simulink model "ofdm_txrx_supermimo"  (Simulink 7.1)
settings: Solver=VariableStepDiscrete  SolverMode=SingleTasking  StartTime=0.0  StopTime=simOnly_simLength  RelTol=1e-3
PostLoadFcn:
  | ofdm_rx_supermimo_init
InitFcn:
  | ofdm_rx_supermimo_init
StartFcn:
  | ofdm_rx_supermimo_init
values from neighbouring files (symbols this model uses):
  Action0_Config = 0* 31*2^18 + 0*2^24 + PHY_AUTORESPONSE_REQ_GOODHDR + PHY_AUTORESPONSE_REQ_GOODPKT + PHY_AUTORESPONSE_REQ_FLAGA    [ofdm_tx_supermimo_init.m]
  Action1_Config = 0* 62*2^18 + PHY_AUTORESPONSE_REQ_GOODHDR + PHY_AUTORESPONSE_REQ_GOODPKT    [ofdm_tx_supermimo_init.m]
  Action2_Config = 0    [ofdm_tx_supermimo_init.m]
  Action3_Config = 0    [ofdm_tx_supermimo_init.m]
  Action4_Config = 0    [ofdm_tx_supermimo_init.m]
  Action5_Config = 0    [ofdm_tx_supermimo_init.m]
  ActionID_AFTransmit = 31    [ofdm_tx_supermimo_init.m]
  ActionID_Disabled = 0    [ofdm_tx_supermimo_init.m]
  ActionID_SetFlagA = 62    [ofdm_tx_supermimo_init.m]
  ActionID_SetFlagB = 61    [ofdm_tx_supermimo_init.m]
  BER_RAM_init_size = 2048    [ofdm_tx_supermimo_init.m]
  CPLength = 16    [ofdm_tx_supermimo_init.m]
  longCorr_coef_i = [-4*real(LTS) 0]    [ofdm_rx_supermimo_init.m]
  longCorr_coef_q = [4*imag(LTS) 0]    [ofdm_rx_supermimo_init.m]
  Match0_Config = 0    [ofdm_tx_supermimo_init.m]
  Match1_Config = 0    [ofdm_tx_supermimo_init.m]
  Match2_Config = 0    [ofdm_tx_supermimo_init.m]
  Match3_Config = 0    [ofdm_tx_supermimo_init.m]
  Match4_Config = 0    [ofdm_tx_supermimo_init.m]
  Match5_Config = 0    [ofdm_tx_supermimo_init.m]
  Match6_Config = 0    [ofdm_tx_supermimo_init.m]
  Match7_Config = 0    [ofdm_tx_supermimo_init.m]
  max_CP_length = 16    [ofdm_tx_supermimo_init.m]
  max_num_baseRateSymbols = 31    [ofdm_tx_supermimo_init.m]
  max_num_subcarriers = 64    [ofdm_tx_supermimo_init.m]
  max_num_trainingSymbols = 15    [ofdm_tx_supermimo_init.m]
  max_numBytes = 16384    [ofdm_tx_supermimo_init.m]
  max_OFDM_symbols = 511    [ofdm_tx_supermimo_init.m]
  modConstellation_qam16 = .75*[1 3 -1 -3]./3    [ofdm_tx_supermimo_init.m]
  modConstellation_qam64 = 0.875*[3 1 5 7 -3 -1 -5 -7]./7    [ofdm_tx_supermimo_init.m]
  modConstellation_qpsk = [1 -1]./sqrt(2)    [ofdm_tx_supermimo_init.m]
  numBaseRateSymbols = ceil(numHeaderBytes / numBytes_BaseRateOFDMSymbol)    [ofdm_tx_supermimo_init.m]
  numSubcarriers = 64    [ofdm_tx_supermimo_init.m]
  numTrainingSymbols = 2    [ofdm_tx_supermimo_init.m]
  pilot_indicies = 7 + ( (64-21) * 2^8) + ( (64-7) * 2^16) + (21 * 2^24)    (= 356068103)    [ofdm_tx_supermimo_init.m]
  pilotValues = hex2dec('A57D') + (2^16 * hex2dec('5A82'))    [ofdm_tx_supermimo_init.m]
  pkt_crossCorr_thresh = 0.7    [ofdm_rx_supermimo_init.m]
  pkt_energy_thresh = 0    [ofdm_rx_supermimo_init.m]
  pktByteNums = numHeaderBytes +  				byteIndex_numPayloadBytes(1)*2^8 +  				byteIndex_numPayloadBytes(2)*2^16 +  				byteIndex_simpleDynModMasks * 2^24    [ofdm_rx_supermimo_init.m]
  PktDet_Delay = 58+32 +      Symbol_Timing_Offset*2^7 +      0*2^16 +      (117* 2^24)    (= 1962935642)    [ofdm_rx_supermimo_init.m]
  pktTiming_count_payloadStart = pktTiming_count_lsStart + pktTiming_ls_num*pktTiming_ls_length    [ofdm_rx_supermimo_init.m]
  preamble = preamble_scale*[shortsyms_10 longsyms_2]    [ofdm_tx_supermimo_init.m]
  preamble_ROM = preamble_I_ROM + preamble_Q_ROM*2^16    [ofdm_tx_supermimo_init.m]
  RAM_init_size = max_numBytes    (= 16384)    [ofdm_tx_supermimo_init.m]
  reg_AF_Tx_Blanking = (320+80) + ( (320+80+79)*2^16)    (= 31392144)    [ofdm_rx_supermimo_init.m]
  reg_AF_Tx_Scaling = 2944    [ofdm_rx_supermimo_init.m]
  reg_Interrupt_PktBuf_Control = reg_InterruptControl +  	1 * 2^16 +  	0 * 2^24    (= 65656)    [ofdm_rx_supermimo_init.m]
  reg_PilotCalcParams = (4 * 2^16) + (minPilotChanMag)    (= 262184.96)    [ofdm_rx_supermimo_init.m]
  regRx_chanEst_minMag = ((0.5)*2^15) + ((0.5 * 2^15)*2^16)    (= 1073758208)    [ofdm_rx_supermimo_init.m]
  regRx_coarseCalcCorrection = round((1.3e-3 - 1.2982e-3)*2^32)    [ofdm_rx_supermimo_init.m]
  regRx_pilotCalcCorrection = round(1.00115 * 2^31)    [ofdm_rx_supermimo_init.m]
  regRx_preCFOoptions = 0 * 2^0 +  	1 * 2^1 +  	0    (= 2)    [ofdm_rx_supermimo_init.m]
  rx_controlBits = 1 * 2  		 + 1 * 4  		 + 0 * 8  		 + 1 * 16 * rx_SISO_Mode  		 + 1 * 32  		 + 1 * 64  		 + 1 * 128  		 + 1 * 256  		 + 1 * 512  		 + 1 * 1024  		 + 1 * 4096  		 + 0 * 16384  		 + 0 * 32768  		 + 1 * 65536  		 + 0 * 131072  		 + 0 * 262144  		 + 1 * 524288  		 + 1 * 1048576 * tx_Alamouti_Mode  		 + 0 * 2097152           + 1 * 33554432  		 + 0 * 4194304  		 + 0 * 8388608  		 + 0 * 16777216  		 + 1 *  ...(+49 chars)    [ofdm_rx_supermimo_init.m]
  Rx_PktDet_LongCorr_Params = LongCorrCounterLoad + 0*2^8 + 0*2^16 + 255*2^24    (= 4278190325)    [ofdm_rx_supermimo_init.m]
  Rx_PktDet_LongCorrThresholds = 8000    [ofdm_rx_supermimo_init.m]
  rx_postEq_scaling = round(rxScaling*2^11) * (1 + 2^16)    [ofdm_rx_supermimo_init.m]
  RxDataScrambling_Seq = [40 198 78 63 82 173 102 245 48 111 172 115 147 230 216 93 72 65 62 2 205 242 122 90 128 83 105 97 73 10 5 252]    [ofdm_tx_supermimo_init.m]
  RxReg_FixedPktLen = 0    [ofdm_rx_supermimo_init.m]
  shortSymbol_time = shortSymbol_time(1:16).'    [ofdm_tx_supermimo_init.m]
  simOnly_numSamples = length(preamble)+( (numSubcarriers+CPLength)*(numTrainingSymbols + numBaseRateSymbols + numFullRateSymbols/2) )    [ofdm_tx_supermimo_init.m]
  train = [train train]    [ofdm_tx_supermimo_init.m]
  tx_controlBits = (antB_preambleShift * 2^4) + (2^1 * tx_Alamouti_Mode) +  	(2^0 * tx_SISO_Mode) + 4*0 + 8*1 + 0*256 + 512*0 + 1024*0 +  	0*hex2dec('1000') + 0*hex2dec('2000') + 0*hex2dec('4000') +  	1*hex2dec('8000') + 1*hex2dec('10000') + hex2dec('20000') +  	16*hex2dec('100000')    [ofdm_tx_supermimo_init.m]
  tx_delays = (50*2^0) + (0*2^8) + (30*2^12)    (= 122930)    [ofdm_tx_supermimo_init.m]
  tx_scaling = (3072) + (12288 * 2^16)    (= 805309440)    [ofdm_tx_supermimo_init.m]
  TxDataScrambling_Seq = [40 198 78 63 82 173 102 245 48 111 172 115 147 230 216 93 72 65 62 2 205 242 122 90 128 83 105 97 73 10 5 252]    [ofdm_tx_supermimo_init.m]
  txfilt_firpm = 0.95*txfilt_firpm./(max(txfilt_firpm))    [ofdm_tx_supermimo_init.m]
  txfilt_intfilt = [0 intfilt(4,4,.8)]    [ofdm_tx_supermimo_init.m]
  txReg_symbolCounts = (2^8 *numBaseRateSymbols ) + numTrainingSymbols    [ofdm_tx_supermimo_init.m]
  TxRx_FFTScaling = (3 * 2^0) +  	(2 * 2^2) +  	(1 * 2^4) +  	(1 * 2^6) +  	(1 * 2^8) +  	(0 * 2^10)    (= 347)    [ofdm_tx_supermimo_init.m]
undefined symbols (not defined in the file or in its folder): AntA, AntB, BaseRate, Down, gcbh, numBuffs, numBytes, Up, xlBlockLoadCallback, xlGetSimulinkPeriod, xlipgetpartsetting
libraries: xbsIndex_r4 x6035, simulink x4, dspsigops x4, commchan3 x2, dspsrcs4 x2, commrflib2 x2
(part 40 of 61)

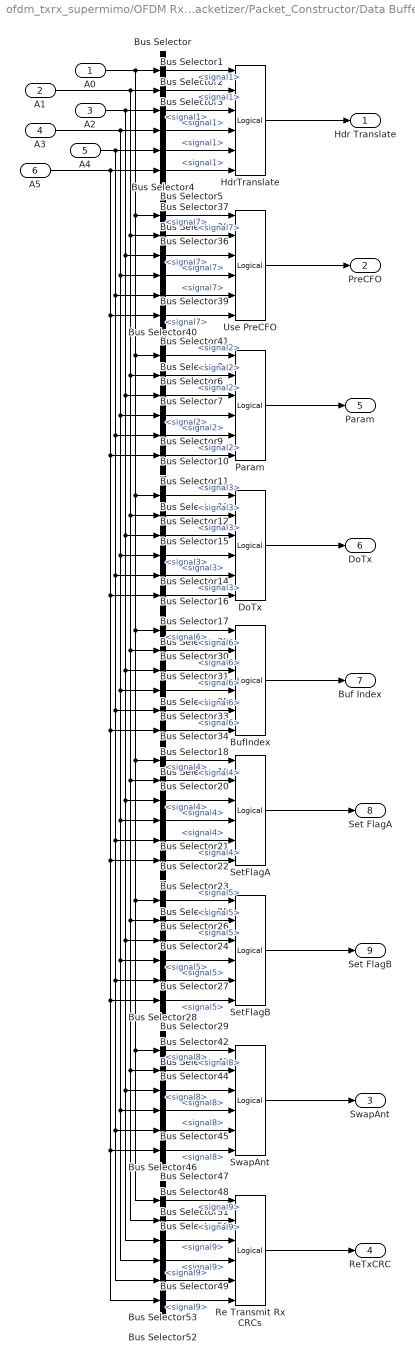
== system ofdm_txrx_supermimo/OFDM Rx MIMO/Equalizer & Packetizer/Packet_Constructor/Data Buffer/AutoResponse Processing/Action Combining ==
  A0 : Inport
  A1 : Inport  Port=2
  A2 : Inport  Port=3
  A3 : Inport  Port=4
  A4 : Inport  Port=5
  A5 : Inport  Port=6
  BufIndex : xbsIndex_r4/Logical  logical_function=OR  inputs=6  en=off  latency=0  precision=Full  arith_type=Unsigned  n_bits=16  bin_pt=0  align_bp=on  dbl_ovrd=off  block_type=logical
  Bus Selector{,1..5} (6 alike) : BusSelector  OutputSignals=signal1
  Bus Selector{6..11} (6 alike) : BusSelector  OutputSignals=signal2
  Bus Selector{12..17} (6 alike) : BusSelector  OutputSignals=signal3
  Bus Selector{18..23} (6 alike) : BusSelector  OutputSignals=signal4
  Bus Selector{24..29} (6 alike) : BusSelector  OutputSignals=signal5
  Bus Selector{30..35} (6 alike) : BusSelector  OutputSignals=signal6
  Bus Selector{36..41} (6 alike) : BusSelector  OutputSignals=signal7
  Bus Selector{42..47} (6 alike) : BusSelector  OutputSignals=signal8
  Bus Selector{48..53} (6 alike) : BusSelector  OutputSignals=signal9
  "DoTx " : xbsIndex_r4/Logical  logical_function=OR  inputs=6  en=off  latency=0  precision=Full  arith_type=Unsigned  n_bits=16  bin_pt=0  align_bp=on  dbl_ovrd=off  block_type=logical
  HdrTranslate : xbsIndex_r4/Logical  logical_function=OR  inputs=6  en=off  latency=0  precision=Full  arith_type=Unsigned  n_bits=16  bin_pt=0  align_bp=on  dbl_ovrd=off  block_type=logical
  "Param " : xbsIndex_r4/Logical  logical_function=OR  inputs=6  en=off  latency=0  precision=Full  arith_type=Unsigned  n_bits=16  bin_pt=0  align_bp=on  dbl_ovrd=off  block_type=logical
  Re Transmit Rx CRCs : xbsIndex_r4/Logical  logical_function=OR  inputs=6  en=off  latency=0  precision=Full  arith_type=Unsigned  n_bits=16  bin_pt=0  align_bp=on  dbl_ovrd=off  block_type=logical
  "SetFlagA " : xbsIndex_r4/Logical  logical_function=OR  inputs=6  en=off  latency=0  precision=Full  arith_type=Unsigned  n_bits=16  bin_pt=0  align_bp=on  dbl_ovrd=off  block_type=logical
  SetFlagB : xbsIndex_r4/Logical  logical_function=OR  inputs=6  en=off  latency=0  precision=Full  arith_type=Unsigned  n_bits=16  bin_pt=0  align_bp=on  dbl_ovrd=off  block_type=logical
  SwapAnt : xbsIndex_r4/Logical  logical_function=OR  inputs=6  en=off  latency=0  precision=Full  arith_type=Unsigned  n_bits=16  bin_pt=0  align_bp=on  dbl_ovrd=off  block_type=logical
  Use PreCFO : xbsIndex_r4/Logical  logical_function=OR  inputs=6  en=off  latency=0  precision=Full  arith_type=Unsigned  n_bits=16  bin_pt=0  align_bp=on  dbl_ovrd=off  block_type=logical
  Hdr Translate : Outport
  PreCFO : Outport  Port=2
  " SwapAnt" : Outport  Port=3
  ReTxCRC : Outport  Port=4
  Param : Outport  Port=5
  DoTx : Outport  Port=6
  Buf Index : Outport  Port=7
  Set FlagA : Outport  Port=8
  Set FlagB : Outport  Port=9
  wires:
  A3/1 -> Bus Selector3/1, Bus Selector9/1, Bus Selector33/1, Bus Selector21/1, Bus Selector27/1, Bus Selector45/1, Bus Selector49/1, Bus Selector14/1, Bus Selector39/1
  A4/1 -> Bus Selector4/1, Bus Selector10/1, Bus Selector34/1, Bus Selector22/1, Bus Selector28/1, Bus Selector46/1, Bus Selector53/1, Bus Selector16/1, Bus Selector40/1
  A5/1 -> Bus Selector5/1, Bus Selector41/1, Bus Selector11/1, Bus Selector17/1, Bus Selector35/1, Bus Selector23/1, Bus Selector29/1, Bus Selector47/1, Bus Selector52/1
  Bus Selector29/1 -> SetFlagB/6  "<signal5>"
  Bus Selector39/1 -> Use PreCFO/4  "<signal7>"
  Bus Selector40/1 -> Use PreCFO/5  "<signal7>"
  Bus Selector41/1 -> Use PreCFO/6  "<signal7>"
  Bus Selector38/1 -> Use PreCFO/3  "<signal7>"
  Bus Selector37/1 -> Use PreCFO/2  "<signal7>"
  Bus Selector36/1 -> Use PreCFO/1  "<signal7>"
  Bus Selector35/1 -> BufIndex/6  "<signal6>"
  Bus Selector34/1 -> BufIndex/5  "<signal6>"
  Bus Selector33/1 -> BufIndex/4  "<signal6>"
  Bus Selector32/1 -> BufIndex/3  "<signal6>"
  Bus Selector31/1 -> BufIndex/2  "<signal6>"
  Bus Selector30/1 -> BufIndex/1  "<signal6>"
  Bus Selector28/1 -> SetFlagB/5  "<signal5>"
  Bus Selector27/1 -> SetFlagB/4  "<signal5>"
  Bus Selector25/1 -> SetFlagB/3  "<signal5>"
  Bus Selector24/1 -> SetFlagB/2  "<signal5>"
  Bus Selector26/1 -> SetFlagB/1  "<signal5>"
  Bus Selector23/1 -> "SetFlagA "/6  "<signal4>"
  Bus Selector22/1 -> "SetFlagA "/5  "<signal4>"
  Bus Selector21/1 -> "SetFlagA "/4  "<signal4>"
  Bus Selector19/1 -> "SetFlagA "/3  "<signal4>"
  Bus Selector18/1 -> "SetFlagA "/2  "<signal4>"
  Bus Selector20/1 -> "SetFlagA "/1  "<signal4>"
  Bus Selector17/1 -> "DoTx "/6  "<signal3>"
  Bus Selector16/1 -> "DoTx "/5  "<signal3>"
  Bus Selector14/1 -> "DoTx "/4  "<signal3>"
  Bus Selector13/1 -> "DoTx "/3  "<signal3>"
  Bus Selector15/1 -> "DoTx "/2  "<signal3>"
  Bus Selector12/1 -> "DoTx "/1  "<signal3>"
  Bus Selector11/1 -> "Param "/6  "<signal2>"
  Bus Selector10/1 -> "Param "/5  "<signal2>"
  Bus Selector9/1 -> "Param "/4  "<signal2>"
  Bus Selector8/1 -> "Param "/3  "<signal2>"
  Bus Selector7/1 -> "Param "/2  "<signal2>"
  Bus Selector6/1 -> "Param "/1  "<signal2>"
  Bus Selector5/1 -> HdrTranslate/6  "<signal1>"
  Bus Selector4/1 -> HdrTranslate/5  "<signal1>"
  Bus Selector3/1 -> HdrTranslate/4  "<signal1>"
  Bus Selector2/1 -> HdrTranslate/3  "<signal1>"
  Bus Selector1/1 -> HdrTranslate/2  "<signal1>"
  Bus Selector/1 -> HdrTranslate/1  "<signal1>"
  A2/1 -> Bus Selector38/1, Bus Selector8/1, Bus Selector32/1, Bus Selector19/1, Bus Selector25/1, Bus Selector43/1, Bus Selector51/1, Bus Selector13/1, Bus Selector2/1
  A1/1 -> Bus Selector37/1, Bus Selector7/1, Bus Selector31/1, Bus Selector18/1, Bus Selector24/1, Bus Selector42/1, Bus Selector48/1, Bus Selector15/1, Bus Selector1/1
  A0/1 -> Bus Selector36/1, Bus Selector30/1, Bus Selector20/1, Bus Selector26/1, Bus Selector44/1, Bus Selector50/1, Bus Selector12/1, Bus Selector6/1, Bus Selector/1
  HdrTranslate/1 -> Hdr Translate/1
  Use PreCFO/1 -> PreCFO/1
  "Param "/1 -> Param/1
  "DoTx "/1 -> DoTx/1
  BufIndex/1 -> Buf Index/1
  "SetFlagA "/1 -> Set FlagA/1
  SetFlagB/1 -> Set FlagB/1
  Bus Selector47/1 -> SwapAnt/6  "<signal8>"
  Bus Selector46/1 -> SwapAnt/5  "<signal8>"
  Bus Selector45/1 -> SwapAnt/4  "<signal8>"
  Bus Selector43/1 -> SwapAnt/3  "<signal8>"
  Bus Selector42/1 -> SwapAnt/2  "<signal8>"
  Bus Selector44/1 -> SwapAnt/1  "<signal8>"
  SwapAnt/1 -> " SwapAnt"/1
  Bus Selector50/1 -> Re Transmit Rx CRCs/1  "<signal9>"
  Re Transmit Rx CRCs/1 -> ReTxCRC/1
  Bus Selector48/1 -> Re Transmit Rx CRCs/2  "<signal9>"
  Bus Selector51/1 -> Re Transmit Rx CRCs/3  "<signal9>"
  Bus Selector49/1 -> Re Transmit Rx CRCs/4  "<signal9>"
  Bus Selector53/1 -> Re Transmit Rx CRCs/5  "<signal9>"
  Bus Selector52/1 -> Re Transmit Rx CRCs/6  "<signal9>"

== system ofdm_txrx_supermimo/OFDM Rx MIMO/Equalizer & Packetizer/Packet_Constructor/Data Buffer/AutoResponse Processing/Action Decoding ==
  Match : Inport
  FlagA : Inport  Port=2
  FlagB : Inport  Port=3
  Action0 : Subsystem
  Action1 : Subsystem
  Action2 : Subsystem
  Action3 : Subsystem
  Action4 : Subsystem
  Action5 : Subsystem
  Bus Creator{,1..5} (6 alike) : BusCreator  Inputs=9
  Concat : xbsIndex_r4/Concat  num_inputs=3  dbl_ovrd=off  block_type=concat
      settings: block_version=10.1.3
  " Action0" : Outport
  " Action1" : Outport  Port=2
  " Action2" : Outport  Port=3
  " Action3" : Outport  Port=4
  " Action4" : Outport  Port=5
  " Action5" : Outport  Port=6
  tag scope: RxAutoReply_MatchStatus=global
  wires:
  Match/1 -> Action0/1(Match), Action4/1(Match), Action5/1(Match), Action3/1(Match), Action2/1(Match), Action1/1(Match), Concat/3
  Action0/1(UseTrans) -> Bus Creator/1
  Action0/2(Param) -> Bus Creator/2
  Action0/3(DoTx) -> Bus Creator/3
  Action0/4(Set_FlagA) -> Bus Creator/4
  Action0/5(Set_FlagB) -> Bus Creator/5
  Bus Creator/1 -> " Action0"/1
  FlagA/1 -> Action0/2(FlagA), Action4/2(FlagA), Action5/2(FlagA), Action3/2(FlagA), Action2/2(FlagA), Action1/2(FlagA), Concat/1
  FlagB/1 -> Action4/3(FlagB), Action5/3(FlagB), Action3/3(FlagB), Action2/3(FlagB), Action1/3(FlagB), Action0/3(FlagB), Concat/2
  Action0/6(TxBufNum) -> Bus Creator/6
  Action0/7(UsePreCFO) -> Bus Creator/7
  Concat/1 -> [RxAutoReply_MatchStatus]
  Action0/8(SwapAnt) -> Bus Creator/8
  Action0/9(ReTxCRC) -> Bus Creator/9
  Action1/1(UseTrans) -> Bus Creator1/1
  Action1/2(Param) -> Bus Creator1/2
  Action1/3(DoTx) -> Bus Creator1/3
  Action1/4(Set_FlagA) -> Bus Creator1/4
  Action1/5(Set_FlagB) -> Bus Creator1/5
  Bus Creator1/1 -> " Action1"/1
  Action1/6(TxBufNum) -> Bus Creator1/6
  Action1/7(UsePreCFO) -> Bus Creator1/7
  Action1/8(SwapAnt) -> Bus Creator1/8
  Action1/9(ReTxCRC) -> Bus Creator1/9
  Action2/1(UseTrans) -> Bus Creator2/1
  Action2/2(Param) -> Bus Creator2/2
  Action2/3(DoTx) -> Bus Creator2/3
  Action2/4(Set_FlagA) -> Bus Creator2/4
  Action2/5(Set_FlagB) -> Bus Creator2/5
  Bus Creator2/1 -> " Action2"/1
  Action2/6(TxBufNum) -> Bus Creator2/6
  Action2/7(UsePreCFO) -> Bus Creator2/7
  Action2/8(SwapAnt) -> Bus Creator2/8
  Action2/9(ReTxCRC) -> Bus Creator2/9
  Action3/1(UseTrans) -> Bus Creator3/1
  Action3/2(Param) -> Bus Creator3/2
  Action3/3(DoTx) -> Bus Creator3/3
  Action3/4(Set_FlagA) -> Bus Creator3/4
  Action3/5(Set_FlagB) -> Bus Creator3/5
  Bus Creator3/1 -> " Action3"/1
  Action3/6(TxBufNum) -> Bus Creator3/6
  Action3/7(UsePreCFO) -> Bus Creator3/7
  Action3/8(SwapAnt) -> Bus Creator3/8
  Action3/9(ReTxCRC) -> Bus Creator3/9
  Action4/1(UseTrans) -> Bus Creator4/1
  Action4/2(Param) -> Bus Creator4/2
  Action4/3(DoTx) -> Bus Creator4/3
  Action4/4(Set_FlagA) -> Bus Creator4/4
  Action4/5(Set_FlagB) -> Bus Creator4/5
  Bus Creator4/1 -> " Action4"/1
  Action4/6(TxBufNum) -> Bus Creator4/6
  Action4/7(UsePreCFO) -> Bus Creator4/7
  Action4/8(SwapAnt) -> Bus Creator4/8
  Action4/9(ReTxCRC) -> Bus Creator4/9
  Action5/1(UseTrans) -> Bus Creator5/1
  Action5/2(Param) -> Bus Creator5/2
  Action5/3(DoTx) -> Bus Creator5/3
  Action5/4(Set_FlagA) -> Bus Creator5/4
  Action5/5(Set_FlagB) -> Bus Creator5/5
  Bus Creator5/1 -> " Action5"/1
  Action5/6(TxBufNum) -> Bus Creator5/6
  Action5/7(UsePreCFO) -> Bus Creator5/7
  Action5/8(SwapAnt) -> Bus Creator5/8
  Action5/9(ReTxCRC) -> Bus Creator5/9

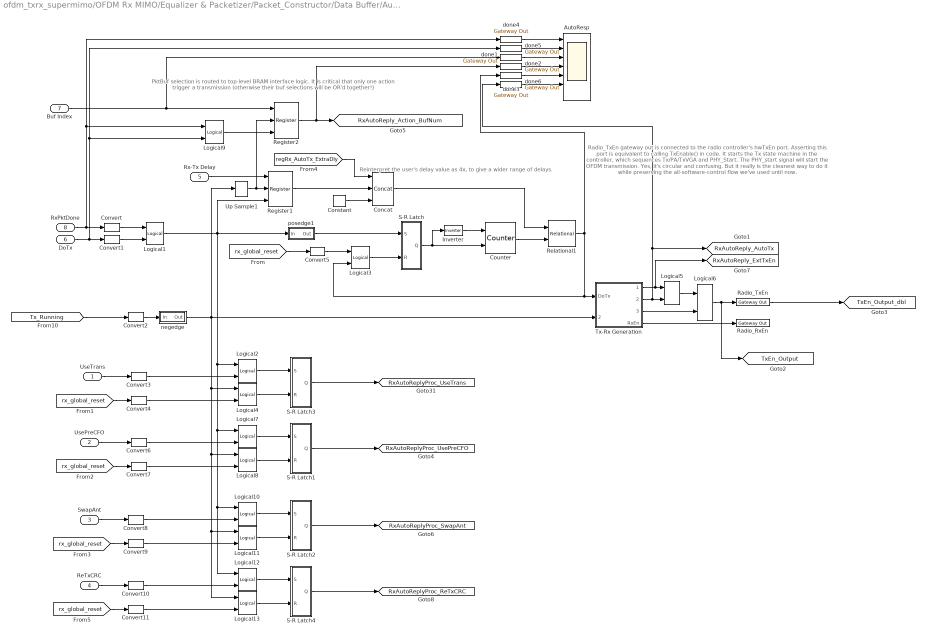
== system ofdm_txrx_supermimo/OFDM Rx MIMO/Equalizer & Packetizer/Packet_Constructor/Data Buffer/AutoResponse Processing/AutoResponse I/O ==
  note: Radio_TxEn gateway out is connected to the radio controller's hwTxEn port.
      | Asserting this port is equivalent to calling TxEnable() in code. It starts the Tx
      | state machine in the controller, which sequences Tx/PA/TxVGA and PHY_Start.
      | The PHY_start signal will start the OFDM transmission.
      |
      | Yes, it's circular and confusing. But it really is the cleanest way to do it while
      | preserving the all-software-control flow we've used until now.
  note: PktBuf selection is routed to top-level
      | BRAM interface logic. It is critical that only
      | one action trigger a transmission (otherwise
      | their buf selections will be OR'd together!)
  note: Reinterpret the user's delay value as 4x,
      | to give a wider range of delays.
  UseTrans : Inport  OutDataType=sfix(16)  OutScaling=2^0
  UsePreCFO : Inport  Port=2  OutDataType=sfix(16)  OutScaling=2^0
  SwapAnt : Inport  Port=3  OutDataType=sfix(16)  OutScaling=2^0
  ReTxCRC : Inport  Port=4  OutDataType=sfix(16)  OutScaling=2^0
  Rx-Tx Delay : Inport  Port=5  OutDataType=sfix(16)  OutScaling=2^0
  DoTx : Inport  Port=6  OutDataType=sfix(16)  OutScaling=2^0
  Buf Index : Inport  Port=7  OutDataType=sfix(16)  OutScaling=2^0
  RxPktDone : Inport  Port=8  OutDataType=sfix(16)  OutScaling=2^0
  AutoResp : Scope  NumInputPorts=7
      settings: TimeRange=392.7801724137931  YMin=-0.5~-0.25~-1~-1~0~0~0  YMax=0.5~0.15~1~1~1~3500~1  SaveName=ScopeData25
  Concat : xbsIndex_r4/Concat  num_inputs=3  dbl_ovrd=off  block_type=concat
      settings: block_version=10.1.3
  Constant : xbsIndex_r4/Constant  arith_type=Unsigned  const=0  n_bits=1  bin_pt=0  explicit_period=off  period=1  equ=P=C  opselect=C  inp2=PCIN>>17  opr=+  inp1=P  carry=CIN  dbl_ovrd=off  block_type=constant
  Convert{,1..11} (12 alike) : xbsIndex_r4/Convert  arith_type=Boolean  n_bits=16  bin_pt=14  quantization=Truncate  overflow=Wrap  latency=0  dbl_ovrd=off  pipeline=off  block_type=convert
      settings: block_version=10.1.3
  Counter : xbsIndex_r4/Counter  cnt_type=Free Running  cnt_to=0  operation=Up  start_count=0  cnt_by_val=1  arith_type=Unsigned  n_bits=14  bin_pt=0  load_pin=off  rst=on  en=on  explicit_period=off  period=4  dbl_ovrd=off  use_behavioral_HDL=off  use_rpm=off  implementation=Fabric  block_type=counter
      settings: block_version=VER_STRING_GOES_HERE
  Inverter : xbsIndex_r4/Inverter  en=off  latency=0  dbl_ovrd=off  block_type=inv
      settings: xl_area=[0 0 0 0 0 0 0]  block_version=8.2
  Logical{1..2,7,9..10,12} (6 alike) : xbsIndex_r4/Logical  logical_function=AND  inputs=2  en=off  latency=0  precision=Full  arith_type=Unsigned  n_bits=16  bin_pt=0  align_bp=on  dbl_ovrd=off  block_type=logical
  Logical{3..6,8,11,13} (7 alike) : xbsIndex_r4/Logical  logical_function=OR  inputs=2  en=off  latency=0  precision=Full  arith_type=Unsigned  n_bits=16  bin_pt=0  align_bp=on  dbl_ovrd=off  block_type=logical
  Radio_RxEn : xbsIndex_r4/Gateway Out  hdl_port=on  timing_constraint=None  locs_specified=off  LOCs={}  block_type=gatewayout
      settings: infoedit="Gateway out block.  Converts Xilinx fixed point inputs into ouputs of type Simulink integer, double, or fixed point.<P><P>Hardware notes:  In hardware these blocks become top level output ports or are discarded, depending on how they are configured."  block_version=10.1.3
  Radio_TxEn : xbsIndex_r4/Gateway Out  hdl_port=on  timing_constraint=None  locs_specified=off  LOCs={}  block_type=gatewayout
      settings: infoedit="Gateway out block.  Converts Xilinx fixed point inputs into ouputs of type Simulink integer, double, or fixed point.<P><P>Hardware notes:  In hardware these blocks become top level output ports or are discarded, depending on how they are configured."  block_version=10.1.3
  Register1 : xbsIndex_r4/Register  init=0  rst=on  en=on  dbl_ovrd=off  block_type=register
      settings: xl_area=[5 10 0 0 0 0 0]  block_version=VER_STRING_GOES_HERE
  Register2 : xbsIndex_r4/Register  init=0  rst=on  en=on  dbl_ovrd=off  block_type=register
      settings: xl_area=[5 10 0 0 0 0 0]  block_version=VER_STRING_GOES_HERE
  Relational1 : xbsIndex_r4/Relational  mode=a<b  en=off  latency=0  dbl_ovrd=off  block_type=relational
      settings: block_version=VER_STRING_GOES_HERE
  S-R Latch : Subsystem
  S-R Latch1 : Subsystem
  S-R Latch2 : Subsystem
  S-R Latch3 : Subsystem
  S-R Latch4 : Subsystem
  Tx-Rx Generation : Subsystem
  Up Sample1 : xbsIndex_r4/Up Sample  sample_ratio=2  copy_samples=on  dbl_ovrd=off  block_type=usamp
      settings: infoedit="Up samples input data.  Inserted values  can be zeros or copies of the most recent input sample.<P><P>Hardware notes: No hardware is needed if inserted values are copies of the input sample; otherwise, a mux and single bit flip-flop are used."  block_version=10.1.3
  done{1..6} (6 alike) : xbsIndex_r4/Gateway Out  hdl_port=off  timing_constraint=None  locs_specified=off  LOCs={}  block_type=gatewayout
      settings: infoedit="Gateway out block.  Converts Xilinx fixed point inputs into ouputs of type Simulink integer, double, or fixed point.<P><P>Hardware notes:  In hardware these blocks become top level output ports or are discarded, depending on how they are configured."  xl_area=[0 0 0 0 1 0 0]  block_version=VER_STRING_GOES_HERE
  negedge : Subsystem
  posedge1 : Subsystem
  tag scope: RxAutoReplyProc_ReTxCRC=global, RxAutoReplyProc_SwapAnt=global, RxAutoReplyProc_UsePreCFO=global, RxAutoReplyProc_UseTrans=global, RxAutoReply_Action_BufNum=global, RxAutoReply_AutoTx=global, RxAutoReply_ExtTxEn=global, TxEn_Output=global, TxEn_Output_dbl=global
  wires:
  S-R Latch/1(Q) -> Counter/2, Inverter/1
  negedge/1(Out) -> Up Sample1/1, Logical4/1, Logical8/1, Logical11/1, Logical13/1, Tx-Rx Generation/2(TxDone)
  [Tx_Running] -> Convert2/1
  posedge1/1(Out) -> S-R Latch/1(S)
  Inverter/1 -> Counter/1
  Tx-Rx Generation/4(RxEn) -> Radio_RxEn/1
  Relational1/1 -> Logical3/2, Tx-Rx Generation/1(DoTx), done3/1
  Logical3/1 -> S-R Latch/2(R)
  [rx_global_reset] -> Convert5/1
  Logical1/1 -> posedge1/1(In), Register1/3, Logical2/1, Logical7/1, Logical10/1, Logical12/1
  Logical2/1 -> S-R Latch3/1(S)
  S-R Latch3/1(Q) -> [RxAutoReplyProc_UseTrans]
  Radio_TxEn/1 -> [TxEn_Output_dbl]
  DoTx/1 -> Convert1/1, Logical9/2, done5/1
  RxPktDone/1 -> Convert/1, Logical9/1, done4/1
  UseTrans/1 -> Convert3/1
  [rx_global_reset] -> Convert4/1
  Logical4/1 -> S-R Latch3/2(R)
  Convert/1 -> Logical1/1
  Convert1/1 -> Logical1/2
  Convert2/1 -> negedge/1(In)
  Convert3/1 -> Logical2/2
  Convert4/1 -> Logical4/2
  Convert5/1 -> Logical3/1
  Tx-Rx Generation/2(AutoTxEn) -> Logical5/2, [RxAutoReply_AutoTx], done6/1
  Logical6/1 -> Radio_TxEn/1, [TxEn_Output]
  Tx-Rx Generation/3(UserTxEn) -> Logical6/2
  Tx-Rx Generation/1(ExtTxEn) -> Logical5/1, [RxAutoReply_ExtTxEn]
  Logical5/1 -> Logical6/1
  Rx-Tx Delay/1 -> Register1/1
  Up Sample1/1 -> Register1/2, Register2/2
  Logical7/1 -> S-R Latch1/1(S)
  S-R Latch1/1(Q) -> [RxAutoReplyProc_UsePreCFO]
  UsePreCFO/1 -> Convert6/1
  [rx_global_reset] -> Convert7/1
  Logical8/1 -> S-R Latch1/2(R)
  Convert6/1 -> Logical7/2
  Convert7/1 -> Logical8/2
  Logical9/1 -> Register2/3
  Buf Index/1 -> Register2/1, done1/1
  Register2/1 -> [RxAutoReply_Action_BufNum], done2/1
  Constant/1 -> Concat/3
  Register1/1 -> Concat/2
  Concat/1 -> Relational1/1
  Counter/1 -> Relational1/2
  SwapAnt/1 -> Convert8/1
  Logical10/1 -> S-R Latch2/1(S)
  Logical11/1 -> S-R Latch2/2(R)
  Convert8/1 -> Logical10/2
  [rx_global_reset] -> Convert9/1
  Convert9/1 -> Logical11/2
  S-R Latch2/1(Q) -> [RxAutoReplyProc_SwapAnt]
  [regRx_AutoTx_ExtraDly] -> Concat/1
  done4/1 -> AutoResp/1
  done5/1 -> AutoResp/2
  done1/1 -> AutoResp/3
  done2/1 -> AutoResp/4
  done3/1 -> AutoResp/5
  done6/1 -> AutoResp/6
  ReTxCRC/1 -> Convert10/1
  Logical12/1 -> S-R Latch4/1(S)
  Logical13/1 -> S-R Latch4/2(R)
  Convert10/1 -> Logical12/2
  [rx_global_reset] -> Convert11/1
  Convert11/1 -> Logical13/2
  S-R Latch4/1(Q) -> [RxAutoReplyProc_ReTxCRC]

== system ofdm_txrx_supermimo/OFDM Rx MIMO/Equalizer & Packetizer/Packet_Constructor/Data Buffer/AutoResponse Processing/Flag A ==
  note: The flag is cleared after every packet reception. The clear is
      | delayed 2 cycles so the matching logic can use the flag set by the
      | previous packet in combination with header/status values
      | from the packet that just finished.
      |
      | The flag is set 1 cycle later if some action asserts the SetFlag input.
  SetFlag : Inport
  PktDone : Inport  Port=2
  Delay : xbsIndex_r4/Delay  en=off  latency=2  dbl_ovrd=off  reg_retiming=off  block_type=delay
  Delay1 : xbsIndex_r4/Delay  en=off  latency=1  dbl_ovrd=off  reg_retiming=off  block_type=delay
  Flag A Latch : Subsystem
  Logical1 : xbsIndex_r4/Logical  logical_function=AND  inputs=2  en=off  latency=0  precision=Full  arith_type=Unsigned  n_bits=16  bin_pt=0  align_bp=on  dbl_ovrd=off  block_type=logical
  Logical2 : xbsIndex_r4/Logical  logical_function=OR  inputs=3  en=off  latency=0  precision=Full  arith_type=Unsigned  n_bits=16  bin_pt=0  align_bp=on  dbl_ovrd=off  block_type=logical
  Flag : Outport
  wires:
  [rx_global_reset] -> Logical2/2
  Logical2/1 -> Flag A Latch/2(R)
  Delay/1 -> Logical2/1, Delay1/1
  Logical1/1 -> Flag A Latch/1(S)
  Flag A Latch/1(Q) -> Flag/1
  PktDone/1 -> Delay/1
  SetFlag/1 -> Logical1/1
  Delay1/1 -> Logical1/2
  [RxReg_ResetFlagA] -> Logical2/3

== system ofdm_txrx_supermimo/OFDM Rx MIMO/Equalizer & Packetizer/Packet_Constructor/Data Buffer/AutoResponse Processing/Flag B ==
  note: The flag is cleared after every packet reception. The clear is
      | delayed 2 cycles so the matching logic can use the flag set by the
      | previous packet in combination with header/status values
      | from the packet that just finished.
      |
      | The flag is set 1 cycle later if some action asserts the SetFlag input.
  SetFlag : Inport
  PktDone : Inport  Port=2
  Delay : xbsIndex_r4/Delay  en=off  latency=2  dbl_ovrd=off  reg_retiming=off  block_type=delay
  Delay1 : xbsIndex_r4/Delay  en=off  latency=1  dbl_ovrd=off  reg_retiming=off  block_type=delay
  Flag B Latch : Subsystem
  Logical1 : xbsIndex_r4/Logical  logical_function=AND  inputs=2  en=off  latency=0  precision=Full  arith_type=Unsigned  n_bits=16  bin_pt=0  align_bp=on  dbl_ovrd=off  block_type=logical
  Logical2 : xbsIndex_r4/Logical  logical_function=OR  inputs=3  en=off  latency=0  precision=Full  arith_type=Unsigned  n_bits=16  bin_pt=0  align_bp=on  dbl_ovrd=off  block_type=logical
  Flag : Outport
  wires:
  [RxReg_ResetFlagB] -> Logical2/3
  Delay1/1 -> Logical1/2
  SetFlag/1 -> Logical1/1
  PktDone/1 -> Delay/1
  Flag B Latch/1(Q) -> Flag/1
  Logical1/1 -> Flag B Latch/1(S)
  Delay/1 -> Delay1/1, Logical2/1
  Logical2/1 -> Flag B Latch/2(R)
  [rx_global_reset] -> Logical2/2

== system ofdm_txrx_supermimo/OFDM Rx MIMO/Equalizer & Packetizer/Packet_Constructor/Data Buffer/AutoResponse Processing/Header Byte Matching ==
  RxByteAddr : Inport  OutDataType=sfix(16)  OutScaling=2^0
  RxByte : Inport  Port=2  OutDataType=sfix(16)  OutScaling=2^0
  RxByteWE : Inport  Port=3  OutDataType=sfix(16)  OutScaling=2^0
  Rst : Inport  Port=4  OutDataType=sfix(16)  OutScaling=2^0
  Concat : xbsIndex_r4/Concat  num_inputs=8  dbl_ovrd=off  block_type=concat
      settings: block_version=10.1.3
  Constant : xbsIndex_r4/Constant  arith_type=Unsigned  const=0  n_bits=1  bin_pt=0  explicit_period=off  period=1  equ=P=C  opselect=C  inp2=PCIN>>17  opr=+  inp1=P  carry=CIN  dbl_ovrd=off  block_type=constant
  Field Slices : Subsystem
  Field Slices1 : Subsystem
  Field Slices2 : Subsystem
  Field Slices3 : Subsystem
  Field Slices4 : Subsystem
  Field Slices5 : Subsystem
  Match0 : Subsystem
  Match1 : Subsystem
  Match2 : Subsystem
  Match3 : Subsystem
  Match4 : Subsystem
  Match5 : Subsystem
  Match_8b : Outport
  wires:
  [regAutoReply_match5] -> Field Slices5/1(Reg 32b)
  Field Slices5/1(Addr) -> Match5/5(Match ByteAddr)
  Field Slices5/2(Len) -> Match5/6(Match Length)
  Field Slices5/3(Val) -> Match5/7(Match Bytes)
  RxByteAddr/1 -> Match0/2(Rx ByteAddr), Match1/2(Rx ByteAddr), Match2/2(Rx ByteAddr), Match3/2(Rx ByteAddr), Match4/2(Rx ByteAddr), Match5/2(Rx ByteAddr)
  RxByte/1 -> Match0/3(Rx Byte), Match1/3(Rx Byte), Match2/3(Rx Byte), Match3/3(Rx Byte), Match4/3(Rx Byte), Match5/3(Rx Byte)
  RxByteWE/1 -> Match0/4(Rx ByteVin), Match1/4(Rx ByteVin), Match2/4(Rx ByteVin), Match3/4(Rx ByteVin), Match4/4(Rx ByteVin), Match5/4(Rx ByteVin)
  Rst/1 -> Match0/1(Rst), Match1/1(Rst), Match2/1(Rst), Match3/1(Rst), Match4/1(Rst), Match5/1(Rst)
  [regAutoReply_match4] -> Field Slices4/1(Reg 32b)
  Field Slices4/1(Addr) -> Match4/5(Match ByteAddr)
  Field Slices4/2(Len) -> Match4/6(Match Length)
  Field Slices4/3(Val) -> Match4/7(Match Bytes)
  [regAutoReply_match3] -> Field Slices3/1(Reg 32b)
  [regAutoReply_match2] -> Field Slices2/1(Reg 32b)
  Field Slices3/1(Addr) -> Match3/5(Match ByteAddr)
  Field Slices3/2(Len) -> Match3/6(Match Length)
  Field Slices3/3(Val) -> Match3/7(Match Bytes)
  Field Slices2/1(Addr) -> Match2/5(Match ByteAddr)
  Field Slices2/2(Len) -> Match2/6(Match Length)
  Field Slices2/3(Val) -> Match2/7(Match Bytes)
  [regAutoReply_match1] -> Field Slices1/1(Reg 32b)
  [regAutoReply_match0] -> Field Slices/1(Reg 32b)
  Field Slices1/1(Addr) -> Match1/5(Match ByteAddr)
  Field Slices1/2(Len) -> Match1/6(Match Length)
  Field Slices1/3(Val) -> Match1/7(Match Bytes)
  Field Slices/1(Addr) -> Match0/5(Match ByteAddr)
  Field Slices/2(Len) -> Match0/6(Match Length)
  Field Slices/3(Val) -> Match0/7(Match Bytes)
  Match5/1(Match) -> Concat/3
  Match4/1(Match) -> Concat/4
  Match3/1(Match) -> Concat/5
  Match2/1(Match) -> Concat/6
  Match1/1(Match) -> Concat/7
  Match0/1(Match) -> Concat/8
  Concat/1 -> Match_8b/1
  Constant/1 -> Concat/1, Concat/2

== system ofdm_txrx_supermimo/OFDM Rx MIMO/Equalizer & Packetizer/Packet_Constructor/Data Buffer/BER Calc/BER Pkt  Buffer ==
  Addr : Inport  OutDataType=sfix(16)  OutScaling=2^0
  ByteComp : Scope  NumInputPorts=3
      settings: TimeRange=6000  YMin=0~0~22.5  YMax=32.5~250~55  SaveName=ScopeData80
  LSB : xbsIndex_r4/Slice  nbits=log2(BER_RAM_init_size)  boolean_output=off  mode=Lower Bit Location + Width  bit1=0  base1=MSB of Input  bit0=0  base0=LSB of Input  dbl_ovrd=off  block_type=slice
      settings: infoedit="Extracts a given range of bits from each input sample and presents it at the output.  The output type is ordinarily unsigned with binary point at zero, but can be Boolean when the slice is one bit wide.<P><P>Hardware notes: In hardware this block costs nothing."  block_version=8.2.01
  Mux : xbsIndex_r4/Mux  inputs=2  en=off  latency=0  precision=Full  arith_type=Unsigned  n_bits=16  bin_pt=14  quantization=Truncate  overflow=Wrap  dbl_ovrd=off  block_type=mux
      settings: block_version=10.1.2
  Read-only (non-PLB) BER Pkt Buffer : Subsystem
  Simulation Multiplexer : xbsIndex_r4/Simulation Multiplexer  sim_sel=1  hw_sel=2  block_type=simmux
      settings: infoedit="Distinguish input subsystems as \"simulation only\" and \"generation only\".  The input specified For Simulation will be used during Simulink simulation.  The input specified For Generation will be used during code generation.  This block will typically be used on the output of a HW Co-Simulation, Black Box, or ModelSim subsystem.<P><P>Hardware Notes: This block costs nothing."  block_version=10.1.3
  done1 : xbsIndex_r4/Gateway Out  hdl_port=off  timing_constraint=None  locs_specified=off  LOCs={}  block_type=gatewayout
      settings: infoedit="Gateway out block.  Converts Xilinx fixed point inputs into ouputs of type Simulink integer, double, or fixed point.<P><P>Hardware notes:  In hardware these blocks become top level output ports or are discarded, depending on how they are configured."  xl_area=[0 0 0 0 1 0 0]  block_version=VER_STRING_GOES_HERE
  done2 : xbsIndex_r4/Gateway Out  hdl_port=off  timing_constraint=None  locs_specified=off  LOCs={}  block_type=gatewayout
      settings: infoedit="Gateway out block.  Converts Xilinx fixed point inputs into ouputs of type Simulink integer, double, or fixed point.<P><P>Hardware notes:  In hardware these blocks become top level output ports or are discarded, depending on how they are configured."  xl_area=[0 0 0 0 1 0 0]  block_version=VER_STRING_GOES_HERE
  done8 : xbsIndex_r4/Gateway Out  hdl_port=off  timing_constraint=None  locs_specified=off  LOCs={}  block_type=gatewayout
      settings: infoedit="Gateway out block.  Converts Xilinx fixed point inputs into ouputs of type Simulink integer, double, or fixed point.<P><P>Hardware notes:  In hardware these blocks become top level output ports or are discarded, depending on how they are configured."  xl_area=[0 0 0 0 1 0 0]  block_version=VER_STRING_GOES_HERE
  ByteOut : Outport  OutDataType=sfix(16)  OutScaling=2^0
  wires:
  Addr/1 -> LSB/1
  LSB/1 -> Read-only (non-PLB) BER Pkt Buffer/1(Addr), done2/1
  Read-only (non-PLB) BER Pkt Buffer/1(Byte) -> Mux/2, done8/1
  [regRx_FlexBERMode] -> Mux/1
  Simulation Multiplexer/1 -> Mux/3, done1/1
  Mux/1 -> ByteOut/1
  done8/1 -> ByteComp/1
  done1/1 -> ByteComp/2
  done2/1 -> ByteComp/3
  [FlexBER_TxPktBuffBtyte_SIM] -> Simulation Multiplexer/1
  [FlexBER_TxPktBuffBtyte_HW] -> Simulation Multiplexer/2

== system ofdm_txrx_supermimo/OFDM Rx MIMO/Equalizer & Packetizer/Packet_Constructor/Data Buffer/BER Calc/BER WEn ==
  note: Calculate the BER of all bytes except checksum bytes.
      | There are up to 6 checksum bytes:
      | Last two bytes of the header
      | Last four bytes of the paylaod IFF there are any payload bytes after the header
  BER_addr : Inport  OutDataType=sfix(16)  OutScaling=2^0
  BER_val : Inport  Port=2  OutDataType=sfix(16)  OutScaling=2^0
  numBytes : Inport  Port=3  OutDataType=sfix(16)  OutScaling=2^0
  AddSub{1..3,6} (4 alike) : xbsIndex_r4/AddSub  mode=Subtraction  use_carryin=off  use_carryout=off  en=off  latency=0  precision=Full  arith_type=Unsigned  n_bits=8  bin_pt=2  quantization=Truncate  overflow=Wrap  dbl_ovrd=off  use_behavioral_HDL=on  pipelined=off  use_rpm=on  hw_selection=Fabric  block_type=addsub
      settings: xl_area=[0, 0, 0, 0, 0, 0, 0]  block_version=VER_STRING_GOES_HERE
  Constant : xbsIndex_r4/Constant  arith_type=Unsigned  const=4  n_bits=3  bin_pt=0  explicit_period=off  period=1  equ=P=C  opselect=C  inp2=PCIN>>17  opr=+  inp1=P  carry=CIN  dbl_ovrd=off  block_type=constant
      settings: block_version=VER_STRING_GOES_HERE
  Constant1 : xbsIndex_r4/Constant  arith_type=Unsigned  const=2  n_bits=2  bin_pt=0  explicit_period=off  period=1  equ=P=C  opselect=C  inp2=PCIN>>17  opr=+  inp1=P  carry=CIN  dbl_ovrd=off  block_type=constant
      settings: block_version=VER_STRING_GOES_HERE
  Constant2 : xbsIndex_r4/Constant  arith_type=Unsigned  const=2  n_bits=2  bin_pt=0  explicit_period=off  period=1  equ=P=C  opselect=C  inp2=PCIN>>17  opr=+  inp1=P  carry=CIN  dbl_ovrd=off  block_type=constant
      settings: block_version=VER_STRING_GOES_HERE
  Constant3 : xbsIndex_r4/Constant  arith_type=Unsigned  const=1  n_bits=2  bin_pt=0  explicit_period=off  period=1  equ=P=C  opselect=C  inp2=PCIN>>17  opr=+  inp1=P  carry=CIN  dbl_ovrd=off  block_type=constant
      settings: block_version=VER_STRING_GOES_HERE
  Inverter : xbsIndex_r4/Inverter  en=off  latency=0  dbl_ovrd=off  block_type=inv
      settings: xl_area=[0, 0, 0, 0, 0, 0, 0]  block_version=VER_STRING_GOES_HERE
  Inverter1 : xbsIndex_r4/Inverter  en=off  latency=0  dbl_ovrd=off  block_type=inv
      settings: xl_area=[0, 0, 0, 0, 0, 0, 0]  block_version=VER_STRING_GOES_HERE
  Inverter2 : xbsIndex_r4/Inverter  en=off  latency=0  dbl_ovrd=off  block_type=inv
      settings: xl_area=[0, 0, 0, 0, 0, 0, 0]  block_version=VER_STRING_GOES_HERE
  Logical1 : xbsIndex_r4/Logical  logical_function=AND  inputs=5  en=off  latency=0  precision=Full  arith_type=Unsigned  n_bits=1  bin_pt=0  align_bp=on  dbl_ovrd=off  block_type=logical
      settings: xl_area=[0, 0, 0, 0, 0, 0, 0]  block_version=VER_STRING_GOES_HERE
  Logical2 : xbsIndex_r4/Logical  logical_function=OR  inputs=2  en=off  latency=0  precision=Full  arith_type=Unsigned  n_bits=1  bin_pt=0  align_bp=on  dbl_ovrd=off  block_type=logical
      settings: xl_area=[0, 0, 0, 0, 0, 0, 0]  block_version=VER_STRING_GOES_HERE
  Logical3 : xbsIndex_r4/Logical  logical_function=AND  inputs=3  en=off  latency=0  precision=Full  arith_type=Unsigned  n_bits=1  bin_pt=0  align_bp=on  dbl_ovrd=off  block_type=logical
      settings: xl_area=[0, 0, 0, 0, 0, 0, 0]  block_version=VER_STRING_GOES_HERE
  Logical4 : xbsIndex_r4/Logical  logical_function=AND  inputs=2  en=off  latency=0  precision=Full  arith_type=Unsigned  n_bits=1  bin_pt=0  align_bp=on  dbl_ovrd=off  block_type=logical
      settings: xl_area=[0, 0, 0, 0, 0, 0, 0]  block_version=VER_STRING_GOES_HERE
  Logical8 : xbsIndex_r4/Logical  logical_function=AND  inputs=3  en=off  latency=0  precision=Full  arith_type=Unsigned  n_bits=1  bin_pt=0  align_bp=on  dbl_ovrd=off  block_type=logical
      settings: xl_area=[0, 0, 0, 0, 0, 0, 0]  block_version=VER_STRING_GOES_HERE
  Relational{,1..2,5} (4 alike) : xbsIndex_r4/Relational  mode=a>b  en=off  latency=0  dbl_ovrd=off  block_type=relational
      settings: xl_area=[0, 0, 0, 0, 0, 0, 0]  block_version=VER_STRING_GOES_HERE
  Relational3 : xbsIndex_r4/Relational  mode=a!=b  en=off  latency=0  dbl_ovrd=off  block_type=relational
      settings: xl_area=[0, 0, 0, 0, 0, 0, 0]  block_version=VER_STRING_GOES_HERE
  Relational4 : xbsIndex_r4/Relational  mode=a!=b  en=off  latency=0  dbl_ovrd=off  block_type=relational
      settings: xl_area=[0, 0, 0, 0, 0, 0, 0]  block_version=VER_STRING_GOES_HERE
  Rx Bytes : Scope  NumInputPorts=5
      settings: TimeRange=1800  YMin=0~50~24~15549.6~-1  YMax=1~275~30~17186.4~1  SaveName=ScopeData3
  done{1..2,6..7,9} (5 alike) : xbsIndex_r4/Gateway Out  hdl_port=off  timing_constraint=None  locs_specified=off  LOCs={}  block_type=gatewayout
      settings: infoedit="Gateway out block.  Converts Xilinx fixed point inputs into ouputs of type Simulink integer, double, or fixed point.<P><P>Hardware notes:  In hardware these blocks become top level output ports or are discarded, depending on how they are configured."  xl_area=[0 0 0 0 1 0 0]  block_version=VER_STRING_GOES_HERE
  Vout : Outport  OutDataType=sfix(16)  OutScaling=2^0
  wires:
  Inverter/1 -> Logical8/3
  BER_val/1 -> Logical8/2
  [rx_global_reset] -> Inverter/1
  Constant/1 -> AddSub6/2
  numBytes/1 -> AddSub6/1, Relational1/1, AddSub1/1, done6/1
  AddSub6/1 -> Relational/1
  BER_addr/1 -> Relational2/2, Relational/2, Relational5/2, Relational3/1, Relational4/1
  Logical8/1 -> Vout/1
  [regTxRx_numHeaderBytes] -> Relational1/2, AddSub2/1, AddSub3/1, Relational5/1, done7/1
  Relational/1 -> Logical1/2
  Relational1/1 -> Inverter1/1, Logical1/5
  Logical1/1 -> Logical2/1, done1/1
  AddSub1/1 -> Relational2/1
  Constant1/1 -> AddSub1/2
  Relational2/1 -> Logical3/3
  Inverter1/1 -> Logical3/2
  Logical3/1 -> Logical2/2
  Logical2/1 -> Logical8/1
  AddSub3/1 -> Relational3/2, done2/1
  AddSub2/1 -> Relational4/2, done9/1
  Relational3/1 -> Logical1/3
  Relational4/1 -> Logical1/4
  done6/1 -> Rx Bytes/1
  done7/1 -> Rx Bytes/2
  done1/1 -> Rx Bytes/3
  done9/1 -> Rx Bytes/5
  done2/1 -> Rx Bytes/4
  Constant3/1 -> AddSub3/2
  Constant2/1 -> AddSub2/2
  [regRx_BERignoreHeader] -> Logical4/1
  Relational5/1 -> Logical4/2
  Logical4/1 -> Inverter2/1
  Inverter2/1 -> Logical1/1, Logical3/1

== system ofdm_txrx_supermimo/OFDM Rx MIMO/Equalizer & Packetizer/Packet_Constructor/Data Buffer/Descrambling & Pkt Buffer/BRAM WEn Decoding ==
  note: FlexBER mode configures the receiver to use
      | the Tx packet buffer as a "golden" packet for
      | BER calculations. In this mode, the receiver
      | does *not* write received packets to the pkt buffer!
  note: Two reasons to allow bytes to be written to the packet buffer:
      | -The PHY is in receive mode, and bytes are being generated by
      | the Rx pipeline.
      | OR
      | -The PHY is in transmit mode, generating random payloads interally
      | but wants to capture those payloads to a packet buffer for offline
      | analysis.
  Byte Addr : Inport  OutDataType=sfix(16)  OutScaling=2^0
  WrEn : Inport  Port=2  OutDataType=sfix(16)  OutScaling=2^0
  3LSB : xbsIndex_r4/Slice  nbits=3  boolean_output=off  mode=Lower Bit Location + Width  bit1=0  base1=MSB of Input  bit0=0  base0=LSB of Input  dbl_ovrd=off  block_type=slice
      settings: infoedit="Extracts a given range of bits from each input sample and presents it at the output.  The output type is ordinarily unsigned with binary point at zero, but can be Boolean when the slice is one bit wide.<P><P>Hardware notes: In hardware this block costs nothing."  block_version=8.2.02
  Concat : xbsIndex_r4/Concat  num_inputs=8  dbl_ovrd=off  block_type=concat
      settings: block_version=8.2.02
  Constant1 : xbsIndex_r4/Constant  arith_type=Unsigned  const=0  n_bits=3  bin_pt=0  explicit_period=off  period=2  equ=P=C  opselect=C  inp2=PCIN>>17  opr=+  inp1=P  carry=CIN  dbl_ovrd=off  block_type=constant
      settings: block_version=8.2.02
  Constant2 : xbsIndex_r4/Constant  arith_type=Unsigned  const=1  n_bits=3  bin_pt=0  explicit_period=off  period=2  equ=P=C  opselect=C  inp2=PCIN>>17  opr=+  inp1=P  carry=CIN  dbl_ovrd=off  block_type=constant
      settings: block_version=8.2.02
  Constant3 : xbsIndex_r4/Constant  arith_type=Unsigned  const=2  n_bits=3  bin_pt=0  explicit_period=off  period=2  equ=P=C  opselect=C  inp2=PCIN>>17  opr=+  inp1=P  carry=CIN  dbl_ovrd=off  block_type=constant
      settings: block_version=8.2.02
  Constant4 : xbsIndex_r4/Constant  arith_type=Unsigned  const=3  n_bits=3  bin_pt=0  explicit_period=off  period=2  equ=P=C  opselect=C  inp2=PCIN>>17  opr=+  inp1=P  carry=CIN  dbl_ovrd=off  block_type=constant
      settings: block_version=8.2.02
  Constant5 : xbsIndex_r4/Constant  arith_type=Unsigned  const=4  n_bits=3  bin_pt=0  explicit_period=off  period=2  equ=P=C  opselect=C  inp2=PCIN>>17  opr=+  inp1=P  carry=CIN  dbl_ovrd=off  block_type=constant
      settings: block_version=8.2.02
  Constant6 : xbsIndex_r4/Constant  arith_type=Unsigned  const=5  n_bits=3  bin_pt=0  explicit_period=off  period=2  equ=P=C  opselect=C  inp2=PCIN>>17  opr=+  inp1=P  carry=CIN  dbl_ovrd=off  block_type=constant
      settings: block_version=8.2.02
  Constant7 : xbsIndex_r4/Constant  arith_type=Unsigned  const=6  n_bits=3  bin_pt=0  explicit_period=off  period=2  equ=P=C  opselect=C  inp2=PCIN>>17  opr=+  inp1=P  carry=CIN  dbl_ovrd=off  block_type=constant
      settings: block_version=8.2.02
  Constant8 : xbsIndex_r4/Constant  arith_type=Unsigned  const=7  n_bits=3  bin_pt=0  explicit_period=off  period=2  equ=P=C  opselect=C  inp2=PCIN>>17  opr=+  inp1=P  carry=CIN  dbl_ovrd=off  block_type=constant
      settings: block_version=8.2.02
  Convert{,1..2,5} (4 alike) : xbsIndex_r4/Convert  arith_type=Unsigned  n_bits=1  bin_pt=0  quantization=Truncate  overflow=Wrap  latency=0  dbl_ovrd=off  pipeline=off  block_type=convert
      settings: block_version=10.1.3
  Convert4 : xbsIndex_r4/Convert  arith_type=Boolean  n_bits=1  bin_pt=0  quantization=Truncate  overflow=Wrap  latency=0  dbl_ovrd=off  pipeline=off  block_type=convert
      settings: block_version=10.1.3
  Inverter : xbsIndex_r4/Inverter  en=off  latency=0  dbl_ovrd=off  block_type=inv
  Inverter1 : xbsIndex_r4/Inverter  en=off  latency=0  dbl_ovrd=off  block_type=inv
  Inverter2 : xbsIndex_r4/Inverter  en=off  latency=0  dbl_ovrd=off  block_type=inv
  Logical{,1..7} (8 alike) : xbsIndex_r4/Logical  logical_function=AND  inputs=2  en=off  latency=0  precision=Full  arith_type=Unsigned  n_bits=16  bin_pt=0  align_bp=on  dbl_ovrd=off  block_type=logical
      settings: block_version=8.2.02
  Logical10 : xbsIndex_r4/Logical  logical_function=OR  inputs=2  en=off  latency=0  precision=Full  arith_type=Unsigned  n_bits=16  bin_pt=0  align_bp=on  dbl_ovrd=off  block_type=logical
  Logical8 : xbsIndex_r4/Logical  logical_function=AND  inputs=4  en=off  latency=0  precision=Full  arith_type=Unsigned  n_bits=16  bin_pt=0  align_bp=on  dbl_ovrd=off  block_type=logical
  Logical9 : xbsIndex_r4/Logical  logical_function=AND  inputs=4  en=off  latency=0  precision=Full  arith_type=Unsigned  n_bits=16  bin_pt=0  align_bp=on  dbl_ovrd=off  block_type=logical
  Relational{,1..7} (8 alike) : xbsIndex_r4/Relational  mode=a=b  en=off  latency=0  dbl_ovrd=off  block_type=relational
      settings: block_version=8.2.02
  Up Sample1 : xbsIndex_r4/Up Sample  sample_ratio=2  copy_samples=on  dbl_ovrd=off  block_type=usamp
      settings: infoedit="Up samples input data.  Inserted values  can be zeros or copies of the most recent input sample.<P><P>Hardware notes: No hardware is needed if inserted values are copies of the input sample; otherwise, a mux and single bit flip-flop are used."  block_version=8.2.02
  Up Sample2 : xbsIndex_r4/Up Sample  sample_ratio=4  copy_samples=on  dbl_ovrd=off  block_type=usamp
      settings: infoedit="Up samples input data.  Inserted values  can be zeros or copies of the most recent input sample.<P><P>Hardware notes: No hardware is needed if inserted values are copies of the input sample; otherwise, a mux and single bit flip-flop are used."  block_version=8.2.02
  Up Sample3 : xbsIndex_r4/Up Sample  sample_ratio=4  copy_samples=on  dbl_ovrd=off  block_type=usamp
      settings: infoedit="Up samples input data.  Inserted values  can be zeros or copies of the most recent input sample.<P><P>Hardware notes: No hardware is needed if inserted values are copies of the input sample; otherwise, a mux and single bit flip-flop are used."  block_version=8.2.02
  Up Sample4 : xbsIndex_r4/Up Sample  sample_ratio=2  copy_samples=on  dbl_ovrd=off  block_type=usamp
      settings: infoedit="Up samples input data.  Inserted values  can be zeros or copies of the most recent input sample.<P><P>Hardware notes: No hardware is needed if inserted values are copies of the input sample; otherwise, a mux and single bit flip-flop are used."  block_version=8.2.02
  Up Sample5 : xbsIndex_r4/Up Sample  sample_ratio=2  copy_samples=on  dbl_ovrd=off  block_type=usamp
      settings: infoedit="Up samples input data.  Inserted values  can be zeros or copies of the most recent input sample.<P><P>Hardware notes: No hardware is needed if inserted values are copies of the input sample; otherwise, a mux and single bit flip-flop are used."  block_version=8.2.02
  ByteEn : Outport  OutDataType=sfix(16)  OutScaling=2^0
  wires:
  Constant1/1 -> Relational/2
  Constant2/1 -> Relational1/2
  Constant3/1 -> Relational2/2
  Constant4/1 -> Relational3/2
  Constant5/1 -> Relational4/2
  Constant6/1 -> Relational5/2
  Constant7/1 -> Relational6/2
  Constant8/1 -> Relational7/2
  Byte Addr/1 -> 3LSB/1
  Relational/1 -> Logical/1
  Relational1/1 -> Logical1/1
  Relational2/1 -> Logical2/1
  Relational3/1 -> Logical3/1
  Relational4/1 -> Logical4/1
  Relational5/1 -> Logical5/1
  Relational6/1 -> Logical6/1
  Relational7/1 -> Logical7/1
  Concat/1 -> ByteEn/1
  3LSB/1 -> Relational/1, Relational1/1, Relational2/1, Relational3/1, Relational4/1, Relational5/1, Relational6/1, Relational7/1
  Logical9/1 -> Logical10/1
  Logical/1 -> Concat/1
  Logical1/1 -> Concat/2
  Logical2/1 -> Concat/3
  Logical3/1 -> Concat/4
  Logical4/1 -> Concat/5
  Logical5/1 -> Concat/6
  Logical6/1 -> Concat/7
  Logical7/1 -> Concat/8
  [regRx_FlexBERMode] -> Inverter/1
  Inverter/1 -> Up Sample1/1
  [regTx_PktRunning] -> Inverter2/1
  Inverter2/1 -> Logical9/3
  [Tx_Running] -> Inverter1/1
  Inverter1/1 -> Up Sample2/1
  Convert/1 -> Logical9/2
  Up Sample1/1 -> Convert1/1
  WrEn/1 -> Convert2/1
  Up Sample2/1 -> Convert/1
  Convert1/1 -> Logical9/1
  Convert4/1 -> Logical/2, Logical1/2, Logical2/2, Logical3/2, Logical4/2, Logical5/2, Logical6/2, Logical7/2
  Logical10/1 -> Convert4/1
  Logical8/1 -> Logical10/2
  Convert2/1 -> Logical9/4, Logical8/1
  [regTx_CaptureRandomPayload] -> Up Sample4/1
  [regTx_RandomPayload] -> Up Sample5/1
  [Tx_Running] -> Up Sample3/1
  Convert5/1 -> Logical8/2
  Up Sample3/1 -> Convert5/1
  Up Sample4/1 -> Logical8/3
  Up Sample5/1 -> Logical8/4
